simulink model "ClimaEgyCoorr"  (R2022b)
settings: Solver=FixedStepDiscrete  StartTime=0.0  StopTime=10.0  FixedStep=1  RelTol=1e-3
settings Model: Creator=blue.ge  LastModifiedBy=blue.ge
settings DataIOCC: SaveFormat=StructureWithTime
settings OptimizationCC: DefaultParameterBehavior=Inlined  EnhancedBackFolding=on  PassReuseOutputArgsAs=Individual arguments  ZeroExternalMemoryAtStartup=off  ZeroInternalMemoryAtStartup=off  OptimizeBlockOrder=speed  DifferentSizesBufferReuse=on  OptimizationCustomize=off  EfficientTunableParamExpr=on
settings DebuggingCC: ParameterTunabilityLossMsg=error
settings ModelReferenceCC: ModelReferenceNumInstancesAllowed=Single
settings SFSimCC: MATLABDynamicMemAlloc=off
settings RTWCC: Description=AUTOSAR  SystemTargetFile=autosar.tlc  GenCodeOnly=on  TemplateMakefile=ert_default_tmf
settings CodeAppCC: ForceParamTrailComments=on  IgnoreCustomStorageClasses=off  OperatorAnnotations=on  SimulinkDataObjDesc=on  SFDataObjDesc=on  InsertBlockDesc=on
settings STFCustomTargetCC: TargetLangStandard=C99 (ISO)  MultiwordLength=256  ParMdlRefBuildCompliant=on  CompOptLevelCompliant=on  IncludeMdlTerminateFcn=off  CombineOutputUpdateFcns=on  SuppressErrorStatus=on  ERTCustomFileBanners=on  SupportNonFinite=off  SupportComplex=off  SupportContinuousTime=off  SupportNonInlinedSFcns=off  RemoveResetFunc=on  MATLABClassNameForMDSCustomization=Simulink.SoftwareTarget.ERTCustomization  AutosarCompliant=on  UseToolchainInfoCompliant=on  LookupTableObjectStructAxisOrder=2,1,3,4,...  LUTObjectStructOrderEvenSpacing=Size,Table,Breakpoints  SystemTargetFile=autosar.tlc
settings ERTTargetCC: TargetLangStandard=C99 (ISO)  ConcurrentExecutionCompliant=off  IncludeMdlTerminateFcn=off  SuppressErrorStatus=on  SupportNonFinite=off  SupportComplex=off  ModelStepFunctionPrototypeControlCompliant=off  CPPClassGenCompliant=off  AutosarCompliant=on  LookupTableObjectStructAxisOrder=2,1,3,4,...  LUTObjectStructOrderEvenSpacing=Size,Table,Breakpoints  InlinedParameterPlacement=NonHierarchical  ERTCustomFileTemplate=example_file_process.tlc

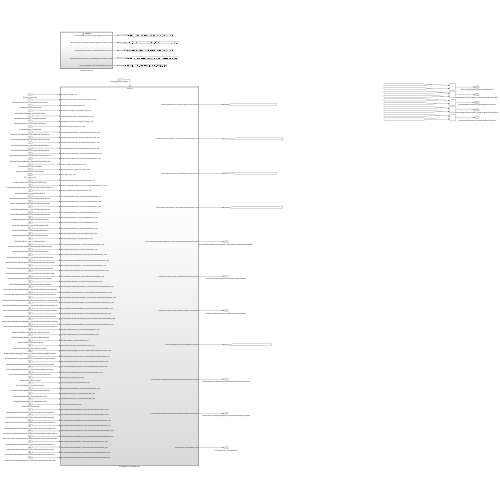
[thumbnail: Simulink's stored preview of the top-level diagram]
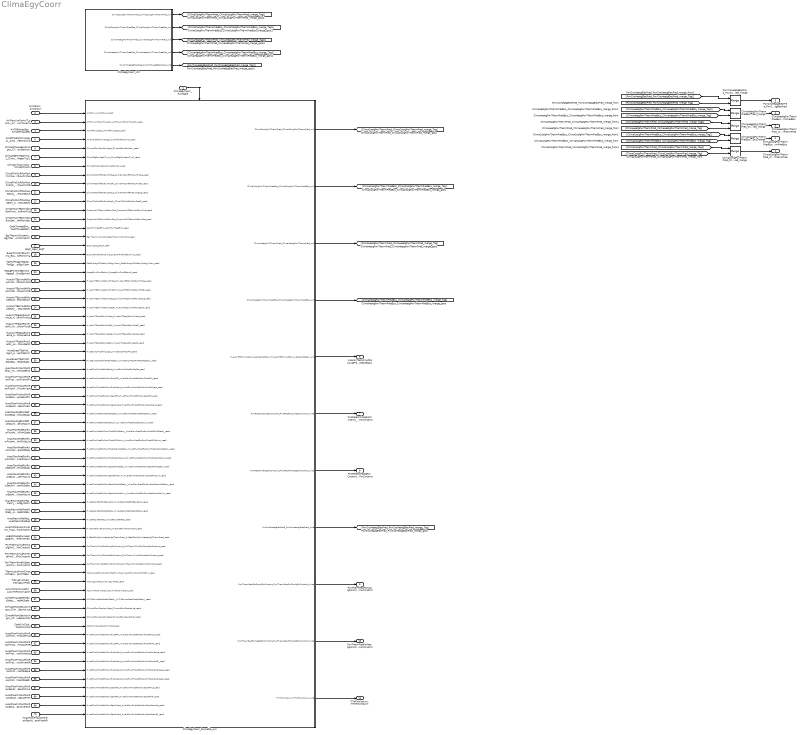
== system ClimaEgyCoorr ==
  ClimaEgyCoorr_Runnable : Inport  SampleTime=1  OutputFunctionCall=on
  AirCdnAvl_AirCdnAvl : Inport  Port=2  OutDataTypeStr=Boolean  PortDimensions=1
  AirFlowLimForAirTLoActv_AirFlowLimForAirTLoActv : Inport  Port=3  OutDataTypeStr=Boolean  PortDimensions=1
  AirTAftHvacDes_AirTAftHvacDes : Inport  Port=4  OutDataTypeStr=T30  PortDimensions=1
  AmbTFildForClimaNgcc_AmbTFildForClima : Inport  Port=5  OutDataTypeStr=T30  PortDimensions=1
  ClimaAtRowSecActvNgcc_ClimaAtRowSecActv : Inport  Port=6  OutDataTypeStr=Boolean  PortDimensions=1
  ClimaCfgForHeatrTyp1_ClimaCfgForHeatrTyp1 : Inport  Port=7  OutDataTypeStr=Enum: ClimaCfgForHeatrTyp1  PortDimensions=1
  ClimaCtrlActvNgcc_ClimaCtrlActv : Inport  Port=8  OutDataTypeStr=Boolean  PortDimensions=1
  ClimaTAtCmftForRowFirstLe_ClimaTAtCmftForRowFirstLe : Inport  Port=9  OutDataTypeStr=T30  PortDimensions=1
  ClimaTAtCmftForRowFirstRi_ClimaTAtCmftForRowFirstRi : Inport  Port=10  OutDataTypeStr=T30  PortDimensions=1
  ClimaTAtCmftForRowSecLe_ClimaTAtCmftForRowSecLe : Inport  Port=11  OutDataTypeStr=T30  PortDimensions=1
  ClimaTAtCmftForRowSecRi_ClimaTAtCmftForRowSecRi : Inport  Port=12  OutDataTypeStr=T30  PortDimensions=1
  CmptmtAirTEstimdAtRowFirst_CmptmtAirTEstimdAtRowFirst : Inport  Port=13  OutDataTypeStr=T30  PortDimensions=1
  CmptmtAirTEstimdAtRowSec_CmptmtAirTEstimdAtRowSec : Inport  Port=14  OutDataTypeStr=T30  PortDimensions=1
  CooltTInHeatElm_CooltTInHeatElm : Inport  Port=15  OutDataTypeStr=T30  PortDimensions=1
  EgyThermInCmptmt_EgyThermInCmptmt : Inport  Port=16  OutDataTypeStr=Egy33  PortDimensions=1
  EngT_Ngcc_EngT : Inport  Port=17  OutDataTypeStr=Bus: EngT  PortDimensions=1  BusOutputAsStruct=on
  EvaprTFrntFildForClima_EvaprTFrntFildForClima : Inport  Port=18  OutDataTypeStr=T30  PortDimensions=1
  FacForProgsToStabyForEgyCoorr_FacForProgsToStabyForEgyCoorr : Inport  Port=19  OutDataTypeStr=Fac30  PortDimensions=1
  HeatgPwrDmdEstimd_HeatgPwrDmdEstimd : Inport  Port=20  OutDataTypeStr=Pwr33  PortDimensions=1
  HvacAirTEstimdAtRowFirstLe_HvacAirTEstimdAtRowFirstLe : Inport  Port=21  OutDataTypeStr=T30  PortDimensions=1
  HvacAirTEstimdAtRowFirstRi_HvacAirTEstimdAtRowFirstRi : Inport  Port=22  OutDataTypeStr=T30  PortDimensions=1
  HvacAirTEstimdAtRowSecLe_HvacAirTEstimdAtRowSecLe : Inport  Port=23  OutDataTypeStr=T30  PortDimensions=1
  HvacAirTEstimdAtRowSecRi_HvacAirTEstimdAtRowSecRi : Inport  Port=24  OutDataTypeStr=T30  PortDimensions=1
  HvacAirTReqAtRowFirstLe_HvacAirTReqAtRowFirstLe : Inport  Port=25  OutDataTypeStr=T30  PortDimensions=1
  HvacAirTReqAtRowFirstRi_HvacAirTReqAtRowFirstRi : Inport  Port=26  OutDataTypeStr=T30  PortDimensions=1
  HvacAirTReqAtRowSecLe_HvacAirTReqAtRowSecLe : Inport  Port=27  OutDataTypeStr=T30  PortDimensions=1
  HvacAirTReqAtRowSecRi_HvacAirTReqAtRowSecRi : Inport  Port=28  OutDataTypeStr=T30  PortDimensions=1
  HvacEvaprTSpFrnt_Ngcc_HvacEvaprTSpFrnt : Inport  Port=29  OutDataTypeStr=HvacEvaprTSp  PortDimensions=1
  HvacEvaprTSpFrntPredAtStaby_HvacEvaprTSpFrntPredAtStaby : Inport  Port=30  OutDataTypeStr=HvacEvaprTSp  PortDimensions=1
  HvacFlowFrntActForDefrst_HvacFlowFrntActForDefrst : Inport  Port=31  OutDataTypeStr=AirFlow30  PortDimensions=1
  HvacFlowFrntActForRowFirstAtFlr_HvacFlowFrntActForRowFirstAtFlr : Inport  Port=32  OutDataTypeStr=AirFlow30  PortDimensions=1
  HvacFlowFrntActForRowFirstAtVent_HvacFlowFrntActForRowFirstAtVent : Inport  Port=33  OutDataTypeStr=AirFlow30  PortDimensions=1
  HvacFlowFrntActForRowSecAtFlr_HvacFlowFrntActForRowSecAtFlr : Inport  Port=34  OutDataTypeStr=AirFlow30  PortDimensions=1
  HvacFlowFrntActForRowSecAtVent_HvacFlowFrntActForRowSecAtVent : Inport  Port=35  OutDataTypeStr=AirFlow30  PortDimensions=1
  HvacFlowPredForDefrstAtStaby_HvacFlowPredForDefrstAtStaby : Inport  Port=36  OutDataTypeStr=AirFlow30  PortDimensions=1
  HvacFlowPredForDefrstNoLim_HvacFlowPredForDefrstNoLim : Inport  Port=37  OutDataTypeStr=AirFlow30  PortDimensions=1
  HvacFlowPredForRowFirstAtFlrAtStaby_HvacFlowPredForRowFirstAtFlrAtStaby : Inport  Port=38  OutDataTypeStr=AirFlow30  PortDimensions=1
  HvacFlowPredForRowFirstAtFlrNoLim_HvacFlowPredForRowFirstAtFlrNoLim : Inport  Port=39  OutDataTypeStr=AirFlow30  PortDimensions=1
  HvacFlowPredForRowFirstAtVentAtStaby_HvacFlowPredForRowFirstAtVentAtStaby : Inport  Port=40  OutDataTypeStr=AirFlow30  PortDimensions=1
  HvacFlowPredForRowFirstAtVentNoLim_HvacFlowPredForRowFirstAtVentNoLim : Inport  Port=41  OutDataTypeStr=AirFlow30  PortDimensions=1
  HvacFlowPredForRowSecAtFlrAtStaby_HvacFlowPredForRowSecAtFlrAtStaby : Inport  Port=42  OutDataTypeStr=AirFlow30  PortDimensions=1
  HvacFlowPredForRowSecAtFlrNoLim_HvacFlowPredForRowSecAtFlrNoLim : Inport  Port=43  OutDataTypeStr=AirFlow30  PortDimensions=1
  HvacFlowPredForRowSecAtVentAtStaby_HvacFlowPredForRowSecAtVentAtStaby : Inport  Port=44  OutDataTypeStr=AirFlow30  PortDimensions=1
  HvacFlowPredForRowSecAtVentNoLim_HvacFlowPredForRowSecAtVentNoLim : Inport  Port=45  OutDataTypeStr=AirFlow30  PortDimensions=1
  HvacRecircRatForEgyCalcn_HvacRecircRatForEgyCalcn : Inport  Port=46  OutDataTypeStr=Perc30  PortDimensions=1
  HvacRecircRatPredAtStaby_HvacRecircRatPredAtStaby : Inport  Port=47  OutDataTypeStr=Perc30  PortDimensions=1
  HvacRecircRatReq_HvacRecircRatReq : Inport  Port=48  OutDataTypeStr=Perc30  PortDimensions=1
  HvacTotFlowForCmptmt_HvacTotFlowForCmptmt : Inport  Port=49  OutDataTypeStr=AirFlow30  PortDimensions=1
  HvBattCoolgPwrHeatgMgrThermPred_HvBattCoolgPwrHeatgMgrThermPred : Inport  Port=50  OutDataTypeStr=Bus: HvBattCoolgPwrHeatgMgrThermPred  PortDimensions=1  BusOutputAsStruct=on
  PwrThermAllwdForCoolgForCmptmt_PwrThermAllwdForCoolgForCmptmt : Inport  Port=51  OutMin=0  OutMax=20460  OutDataTypeStr=Pwr3  PortDimensions=1
  PwrThermAllwdForHeatForCmptmt_PwrThermAllwdForHeatForCmptmt : Inport  Port=52  OutMin=0  OutMax=20460  OutDataTypeStr=Pwr3  PortDimensions=1
  PwrThermDmdAtStabyForCmptmt_PwrThermDmdAtStabyForCmptmt : Inport  Port=53  OutDataTypeStr=Pwr33  PortDimensions=1
  ThermLossFromCmptmtToEnv_ThermLossFromCmptmtToEnv : Inport  Port=54  OutDataTypeStr=Pwr33  PortDimensions=1
  TiDrvgCycPred_TiDrvgCycPred : Inport  Port=55  OutDataTypeStr=Ti43  PortDimensions=1
  ActvnTiForClimaCtrl_ActvnTiForClimaCtrl : Inport  Port=56  OutDataTypeStr=Ti33  PortDimensions=1
  AirTAftHvacDesPredAtStaby_AirTAftHvacDesPredAtStaby : Inport  Port=57  OutDataTypeStr=T30  PortDimensions=1
  ClimaAtRowSecActvNgcc_ClimaAtRowSecActvLe : Inport  Port=58  OutDataTypeStr=Boolean  PortDimensions=1
  ClimaAtRowSecActvNgcc_ClimaAtRowSecActvRi : Inport  Port=59  OutDataTypeStr=Boolean  PortDimensions=1
  CooltVlvClsd_CooltVlvClsd : Inport  Port=60  OutDataTypeStr=Boolean  PortDimensions=1
  HvacFlowFrntActForRowFirstAtFlr_HvacFlowFrntActForRowFirstAtFlrLe : Inport  Port=61  OutDataTypeStr=AirFlow30  PortDimensions=1
  HvacFlowFrntActForRowFirstAtFlr_HvacFlowFrntActForRowFirstAtFlrRi : Inport  Port=62  OutDataTypeStr=AirFlow30  PortDimensions=1
  HvacFlowFrntActForRowFirstAtVent_HvacFlowFrntActForRowFirstAtVentLe : Inport  Port=63  OutDataTypeStr=AirFlow30  PortDimensions=1
  HvacFlowFrntActForRowFirstAtVent_HvacFlowFrntActForRowFirstAtVentRi : Inport  Port=64  OutDataTypeStr=AirFlow30  PortDimensions=1
  HvacFlowFrntActForRowFirstAtVent_HvacFlowFrntActForRowFirstAtVentSideLe : Inport  Port=65  OutDataTypeStr=AirFlow30  PortDimensions=1
  HvacFlowFrntActForRowFirstAtVent_HvacFlowFrntActForRowFirstAtVentSideRi : Inport  Port=66  OutDataTypeStr=AirFlow30  PortDimensions=1
  HvacFlowFrntActForRowSecAtFlr_HvacFlowFrntActForRowSecAtFlrLe : Inport  Port=67  OutDataTypeStr=AirFlow30  PortDimensions=1
  HvacFlowFrntActForRowSecAtFlr_HvacFlowFrntActForRowSecAtFlrRi : Inport  Port=68  OutDataTypeStr=AirFlow30  PortDimensions=1
  HvacFlowFrntActForRowSecAtVent_HvacFlowFrntActForRowSecAtVentLe : Inport  Port=69  OutDataTypeStr=AirFlow30  PortDimensions=1
  HvacFlowFrntActForRowSecAtVent_HvacFlowFrntActForRowSecAtVentRi : Inport  Port=70  OutDataTypeStr=AirFlow30  PortDimensions=1
  ClimaCoolgPwrThermPredEco_ClimaCoolgPwrThermPredEco_merge : Merge
  ClimaCoolgPwrThermPred_ClimaCoolgPwrThermPred_merge : Merge
  ClimaEgyCoorr_Init : Subsystem
  ClimaEgyCoorr_Runnable_sys : Subsystem
  ClimaHeatgPwrThermPredEco_ClimaHeatgPwrThermPredEco_merge : Merge
  ClimaHeatgPwrThermPred_ClimaHeatgPwrThermPred_merge : Merge
  PwrCnsHeatgElecPred_PwrCnsHeatgElecPred_merge : Merge
  ClimaCoolgPwrThermPred_ClimaCoolgPwrThermPred : Outport  OutDataTypeStr=Bus: ClimaCoolgPwrThermPred  PortDimensions=1  BusOutputAsStruct=on
  ClimaCoolgPwrThermPredEco_ClimaCoolgPwrThermPredEco : Outport  Port=2  OutDataTypeStr=Bus: ClimaCoolgPwrThermPredEcoRec  PortDimensions=1  BusOutputAsStruct=on
  ClimaHeatgPwrThermPred_ClimaHeatgPwrThermPred : Outport  Port=3  OutDataTypeStr=Bus: ClimaHeatgPwrThermPred  PortDimensions=1  BusOutputAsStruct=on
  ClimaHeatgPwrThermPredEco_ClimaHeatgPwrThermPredEco : Outport  Port=4  OutDataTypeStr=Bus: ClimaHeatgPwrThermPredEcoRec  PortDimensions=1  BusOutputAsStruct=on
  PwrCnsHeatgElecPred_PwrCnsHeatgElecPred : Outport  Port=5  OutDataTypeStr=Bus: PwrCnsHeatgElecPred  PortDimensions=1  BusOutputAsStruct=on
  HvacAirTEstimnAtRowAvgPredAtStaby_HvacAirTEstimnAtRowAvgPredAtStaby : Outport  Port=6  OutDataTypeStr=T30  PortDimensions=1
  PrioReqForCoolgForCmptmt_PrioReqForCoolgForCmptmt : Outport  Port=7  OutDataTypeStr=Enum: LvlOfPrioTyp  PortDimensions=1
  PrioReqForHeatgForCmptmt_PrioReqForHeatgForCmptmt : Outport  Port=8  OutDataTypeStr=Enum: LvlOfPrioTyp  PortDimensions=1
  PwrThermReqForCoolgForCmptmt_PwrThermReqForCoolgForCmptmt : Outport  Port=9  OutMin=0  OutMax=20460  OutDataTypeStr=Pwr3  PortDimensions=1
  PwrThermReqForHeatgForCmptmt_PwrThermReqForHeatgForCmptmt : Outport  Port=10  OutMin=0  OutMax=20460  OutDataTypeStr=Pwr3  PortDimensions=1
  TiToFlowNoLim_TiToFlowNoLim : Outport  Port=11  OutDataTypeStr=Ti33  PortDimensions=1
  wires:
  AirCdnAvl_AirCdnAvl/1 -> ClimaEgyCoorr_Runnable_sys/1(AirCdnAvl_AirCdnAvl_read)
  AirFlowLimForAirTLoActv_AirFlowLimForAirTLoActv/1 -> ClimaEgyCoorr_Runnable_sys/2(AirFlowLimForAirTLoActv_AirFlowLimForAirTLoActv_read)
  AirTAftHvacDes_AirTAftHvacDes/1 -> ClimaEgyCoorr_Runnable_sys/3(AirTAftHvacDes_AirTAftHvacDes_read)
  AmbTFildForClimaNgcc_AmbTFildForClima/1 -> ClimaEgyCoorr_Runnable_sys/4(AmbTFildForClimaNgcc_AmbTFildForClima_read)
  ClimaAtRowSecActvNgcc_ClimaAtRowSecActv/1 -> ClimaEgyCoorr_Runnable_sys/5(ClimaAtRowSecActvNgcc_ClimaAtRowSecActv_read)
  ClimaCfgForHeatrTyp1_ClimaCfgForHeatrTyp1/1 -> ClimaEgyCoorr_Runnable_sys/6(ClimaCfgForHeatrTyp1_ClimaCfgForHeatrTyp1_read)
  ClimaCtrlActvNgcc_ClimaCtrlActv/1 -> ClimaEgyCoorr_Runnable_sys/7(ClimaCtrlActvNgcc_ClimaCtrlActv_read)
  ClimaTAtCmftForRowFirstLe_ClimaTAtCmftForRowFirstLe/1 -> ClimaEgyCoorr_Runnable_sys/8(ClimaTAtCmftForRowFirstLe_ClimaTAtCmftForRowFirstLe_read)
  ClimaTAtCmftForRowFirstRi_ClimaTAtCmftForRowFirstRi/1 -> ClimaEgyCoorr_Runnable_sys/9(ClimaTAtCmftForRowFirstRi_ClimaTAtCmftForRowFirstRi_read)
  ClimaTAtCmftForRowSecLe_ClimaTAtCmftForRowSecLe/1 -> ClimaEgyCoorr_Runnable_sys/10(ClimaTAtCmftForRowSecLe_ClimaTAtCmftForRowSecLe_read)
  ClimaTAtCmftForRowSecRi_ClimaTAtCmftForRowSecRi/1 -> ClimaEgyCoorr_Runnable_sys/11(ClimaTAtCmftForRowSecRi_ClimaTAtCmftForRowSecRi_read)
  CmptmtAirTEstimdAtRowFirst_CmptmtAirTEstimdAtRowFirst/1 -> ClimaEgyCoorr_Runnable_sys/12(CmptmtAirTEstimdAtRowFirst_CmptmtAirTEstimdAtRowFirst_read)
  CmptmtAirTEstimdAtRowSec_CmptmtAirTEstimdAtRowSec/1 -> ClimaEgyCoorr_Runnable_sys/13(CmptmtAirTEstimdAtRowSec_CmptmtAirTEstimdAtRowSec_read)
  CooltTInHeatElm_CooltTInHeatElm/1 -> ClimaEgyCoorr_Runnable_sys/14(CooltTInHeatElm_CooltTInHeatElm_read)
  EgyThermInCmptmt_EgyThermInCmptmt/1 -> ClimaEgyCoorr_Runnable_sys/15(EgyThermInCmptmt_EgyThermInCmptmt_read)
  EngT_Ngcc_EngT/1 -> ClimaEgyCoorr_Runnable_sys/16(EngT_Ngcc_EngT_read)
  EvaprTFrntFildForClima_EvaprTFrntFildForClima/1 -> ClimaEgyCoorr_Runnable_sys/17(EvaprTFrntFildForClima_EvaprTFrntFildForClima_read)
  FacForProgsToStabyForEgyCoorr_FacForProgsToStabyForEgyCoorr/1 -> ClimaEgyCoorr_Runnable_sys/18(FacForProgsToStabyForEgyCoorr_FacForProgsToStabyForEgyCoorr_read)
  HeatgPwrDmdEstimd_HeatgPwrDmdEstimd/1 -> ClimaEgyCoorr_Runnable_sys/19(HeatgPwrDmdEstimd_HeatgPwrDmdEstimd_read)
  HvacAirTEstimdAtRowFirstLe_HvacAirTEstimdAtRowFirstLe/1 -> ClimaEgyCoorr_Runnable_sys/20(HvacAirTEstimdAtRowFirstLe_HvacAirTEstimdAtRowFirstLe_read)
  HvacAirTEstimdAtRowFirstRi_HvacAirTEstimdAtRowFirstRi/1 -> ClimaEgyCoorr_Runnable_sys/21(HvacAirTEstimdAtRowFirstRi_HvacAirTEstimdAtRowFirstRi_read)
  HvacAirTEstimdAtRowSecLe_HvacAirTEstimdAtRowSecLe/1 -> ClimaEgyCoorr_Runnable_sys/22(HvacAirTEstimdAtRowSecLe_HvacAirTEstimdAtRowSecLe_read)
  HvacAirTEstimdAtRowSecRi_HvacAirTEstimdAtRowSecRi/1 -> ClimaEgyCoorr_Runnable_sys/23(HvacAirTEstimdAtRowSecRi_HvacAirTEstimdAtRowSecRi_read)
  HvacAirTReqAtRowFirstLe_HvacAirTReqAtRowFirstLe/1 -> ClimaEgyCoorr_Runnable_sys/24(HvacAirTReqAtRowFirstLe_HvacAirTReqAtRowFirstLe_read)
  HvacAirTReqAtRowFirstRi_HvacAirTReqAtRowFirstRi/1 -> ClimaEgyCoorr_Runnable_sys/25(HvacAirTReqAtRowFirstRi_HvacAirTReqAtRowFirstRi_read)
  HvacAirTReqAtRowSecLe_HvacAirTReqAtRowSecLe/1 -> ClimaEgyCoorr_Runnable_sys/26(HvacAirTReqAtRowSecLe_HvacAirTReqAtRowSecLe_read)
  HvacAirTReqAtRowSecRi_HvacAirTReqAtRowSecRi/1 -> ClimaEgyCoorr_Runnable_sys/27(HvacAirTReqAtRowSecRi_HvacAirTReqAtRowSecRi_read)
  HvacEvaprTSpFrnt_Ngcc_HvacEvaprTSpFrnt/1 -> ClimaEgyCoorr_Runnable_sys/28(HvacEvaprTSpFrnt_Ngcc_HvacEvaprTSpFrnt_read)
  HvacEvaprTSpFrntPredAtStaby_HvacEvaprTSpFrntPredAtStaby/1 -> ClimaEgyCoorr_Runnable_sys/29(HvacEvaprTSpFrntPredAtStaby_HvacEvaprTSpFrntPredAtStaby_read)
  HvacFlowFrntActForDefrst_HvacFlowFrntActForDefrst/1 -> ClimaEgyCoorr_Runnable_sys/30(HvacFlowFrntActForDefrst_HvacFlowFrntActForDefrst_read)
  HvacFlowFrntActForRowFirstAtFlr_HvacFlowFrntActForRowFirstAtFlr/1 -> ClimaEgyCoorr_Runnable_sys/31(HvacFlowFrntActForRowFirstAtFlr_HvacFlowFrntActForRowFirstAtFlr_read)
  HvacFlowFrntActForRowFirstAtVent_HvacFlowFrntActForRowFirstAtVent/1 -> ClimaEgyCoorr_Runnable_sys/32(HvacFlowFrntActForRowFirstAtVent_HvacFlowFrntActForRowFirstAtVent_read)
  HvacFlowFrntActForRowSecAtFlr_HvacFlowFrntActForRowSecAtFlr/1 -> ClimaEgyCoorr_Runnable_sys/33(HvacFlowFrntActForRowSecAtFlr_HvacFlowFrntActForRowSecAtFlr_read)
  HvacFlowFrntActForRowSecAtVent_HvacFlowFrntActForRowSecAtVent/1 -> ClimaEgyCoorr_Runnable_sys/34(HvacFlowFrntActForRowSecAtVent_HvacFlowFrntActForRowSecAtVent_read)
  HvacFlowPredForDefrstAtStaby_HvacFlowPredForDefrstAtStaby/1 -> ClimaEgyCoorr_Runnable_sys/35(HvacFlowPredForDefrstAtStaby_HvacFlowPredForDefrstAtStaby_read)
  HvacFlowPredForDefrstNoLim_HvacFlowPredForDefrstNoLim/1 -> ClimaEgyCoorr_Runnable_sys/36(HvacFlowPredForDefrstNoLim_HvacFlowPredForDefrstNoLim_read)
  HvacFlowPredForRowFirstAtFlrAtStaby_HvacFlowPredForRowFirstAtFlrAtStaby/1 -> ClimaEgyCoorr_Runnable_sys/37(HvacFlowPredForRowFirstAtFlrAtStaby_HvacFlowPredForRowFirstAtFlrAtStaby_read)
  HvacFlowPredForRowFirstAtFlrNoLim_HvacFlowPredForRowFirstAtFlrNoLim/1 -> ClimaEgyCoorr_Runnable_sys/38(HvacFlowPredForRowFirstAtFlrNoLim_HvacFlowPredForRowFirstAtFlrNoLim_read)
  HvacFlowPredForRowFirstAtVentAtStaby_HvacFlowPredForRowFirstAtVentAtStaby/1 -> ClimaEgyCoorr_Runnable_sys/39(HvacFlowPredForRowFirstAtVentAtStaby_HvacFlowPredForRowFirstAtVentAtStaby_read)
  HvacFlowPredForRowFirstAtVentNoLim_HvacFlowPredForRowFirstAtVentNoLim/1 -> ClimaEgyCoorr_Runnable_sys/40(HvacFlowPredForRowFirstAtVentNoLim_HvacFlowPredForRowFirstAtVentNoLim_read)
  HvacFlowPredForRowSecAtFlrAtStaby_HvacFlowPredForRowSecAtFlrAtStaby/1 -> ClimaEgyCoorr_Runnable_sys/41(HvacFlowPredForRowSecAtFlrAtStaby_HvacFlowPredForRowSecAtFlrAtStaby_read)
  HvacFlowPredForRowSecAtFlrNoLim_HvacFlowPredForRowSecAtFlrNoLim/1 -> ClimaEgyCoorr_Runnable_sys/42(HvacFlowPredForRowSecAtFlrNoLim_HvacFlowPredForRowSecAtFlrNoLim_read)
  HvacFlowPredForRowSecAtVentAtStaby_HvacFlowPredForRowSecAtVentAtStaby/1 -> ClimaEgyCoorr_Runnable_sys/43(HvacFlowPredForRowSecAtVentAtStaby_HvacFlowPredForRowSecAtVentAtStaby_read)
  HvacFlowPredForRowSecAtVentNoLim_HvacFlowPredForRowSecAtVentNoLim/1 -> ClimaEgyCoorr_Runnable_sys/44(HvacFlowPredForRowSecAtVentNoLim_HvacFlowPredForRowSecAtVentNoLim_read)
  HvacRecircRatForEgyCalcn_HvacRecircRatForEgyCalcn/1 -> ClimaEgyCoorr_Runnable_sys/45(HvacRecircRatForEgyCalcn_HvacRecircRatForEgyCalcn_read)
  HvacRecircRatPredAtStaby_HvacRecircRatPredAtStaby/1 -> ClimaEgyCoorr_Runnable_sys/46(HvacRecircRatPredAtStaby_HvacRecircRatPredAtStaby_read)
  HvacRecircRatReq_HvacRecircRatReq/1 -> ClimaEgyCoorr_Runnable_sys/47(HvacRecircRatReq_HvacRecircRatReq_read)
  HvacTotFlowForCmptmt_HvacTotFlowForCmptmt/1 -> ClimaEgyCoorr_Runnable_sys/48(HvacTotFlowForCmptmt_HvacTotFlowForCmptmt_read)
  HvBattCoolgPwrHeatgMgrThermPred_HvBattCoolgPwrHeatgMgrThermPred/1 -> ClimaEgyCoorr_Runnable_sys/49(HvBattCoolgPwrHeatgMgrThermPred_HvBattCoolgPwrHeatgMgrThermPred_read)
  PwrThermAllwdForCoolgForCmptmt_PwrThermAllwdForCoolgForCmptmt/1 -> ClimaEgyCoorr_Runnable_sys/50(PwrThermAllwdForCoolgForCmptmt_PwrThermAllwdForCoolgForCmptmt_read)
  PwrThermAllwdForHeatForCmptmt_PwrThermAllwdForHeatForCmptmt/1 -> ClimaEgyCoorr_Runnable_sys/51(PwrThermAllwdForHeatForCmptmt_PwrThermAllwdForHeatForCmptmt_read)
  PwrThermDmdAtStabyForCmptmt_PwrThermDmdAtStabyForCmptmt/1 -> ClimaEgyCoorr_Runnable_sys/52(PwrThermDmdAtStabyForCmptmt_PwrThermDmdAtStabyForCmptmt_read)
  ThermLossFromCmptmtToEnv_ThermLossFromCmptmtToEnv/1 -> ClimaEgyCoorr_Runnable_sys/53(ThermLossFromCmptmtToEnv_ThermLossFromCmptmtToEnv_read)
  TiDrvgCycPred_TiDrvgCycPred/1 -> ClimaEgyCoorr_Runnable_sys/54(TiDrvgCycPred_TiDrvgCycPred_read)
  ActvnTiForClimaCtrl_ActvnTiForClimaCtrl/1 -> ClimaEgyCoorr_Runnable_sys/55(ActvnTiForClimaCtrl_ActvnTiForClimaCtrl_read)
  AirTAftHvacDesPredAtStaby_AirTAftHvacDesPredAtStaby/1 -> ClimaEgyCoorr_Runnable_sys/56(AirTAftHvacDesPredAtStaby_AirTAftHvacDesPredAtStaby_read)
  ClimaAtRowSecActvNgcc_ClimaAtRowSecActvLe/1 -> ClimaEgyCoorr_Runnable_sys/57(ClimaAtRowSecActvNgcc_ClimaAtRowSecActvLe_read)
  ClimaAtRowSecActvNgcc_ClimaAtRowSecActvRi/1 -> ClimaEgyCoorr_Runnable_sys/58(ClimaAtRowSecActvNgcc_ClimaAtRowSecActvRi_read)
  CooltVlvClsd_CooltVlvClsd/1 -> ClimaEgyCoorr_Runnable_sys/59(CooltVlvClsd_CooltVlvClsd_read)
  HvacFlowFrntActForRowFirstAtFlr_HvacFlowFrntActForRowFirstAtFlrLe/1 -> ClimaEgyCoorr_Runnable_sys/60(HvacFlowFrntActForRowFirstAtFlr_HvacFlowFrntActForRowFirstAtFlrLe_read)
  HvacFlowFrntActForRowFirstAtFlr_HvacFlowFrntActForRowFirstAtFlrRi/1 -> ClimaEgyCoorr_Runnable_sys/61(HvacFlowFrntActForRowFirstAtFlr_HvacFlowFrntActForRowFirstAtFlrRi_read)
  HvacFlowFrntActForRowFirstAtVent_HvacFlowFrntActForRowFirstAtVentLe/1 -> ClimaEgyCoorr_Runnable_sys/62(HvacFlowFrntActForRowFirstAtVent_HvacFlowFrntActForRowFirstAtVentLe_read)
  HvacFlowFrntActForRowFirstAtVent_HvacFlowFrntActForRowFirstAtVentRi/1 -> ClimaEgyCoorr_Runnable_sys/63(HvacFlowFrntActForRowFirstAtVent_HvacFlowFrntActForRowFirstAtVentRi_read)
  HvacFlowFrntActForRowFirstAtVent_HvacFlowFrntActForRowFirstAtVentSideLe/1 -> ClimaEgyCoorr_Runnable_sys/64(HvacFlowFrntActForRowFirstAtVent_HvacFlowFrntActForRowFirstAtVentSideLe_read)
  HvacFlowFrntActForRowFirstAtVent_HvacFlowFrntActForRowFirstAtVentSideRi/1 -> ClimaEgyCoorr_Runnable_sys/65(HvacFlowFrntActForRowFirstAtVent_HvacFlowFrntActForRowFirstAtVentSideRi_read)
  HvacFlowFrntActForRowSecAtFlr_HvacFlowFrntActForRowSecAtFlrLe/1 -> ClimaEgyCoorr_Runnable_sys/66(HvacFlowFrntActForRowSecAtFlr_HvacFlowFrntActForRowSecAtFlrLe_read)
  HvacFlowFrntActForRowSecAtFlr_HvacFlowFrntActForRowSecAtFlrRi/1 -> ClimaEgyCoorr_Runnable_sys/67(HvacFlowFrntActForRowSecAtFlr_HvacFlowFrntActForRowSecAtFlrRi_read)
  HvacFlowFrntActForRowSecAtVent_HvacFlowFrntActForRowSecAtVentLe/1 -> ClimaEgyCoorr_Runnable_sys/68(HvacFlowFrntActForRowSecAtVent_HvacFlowFrntActForRowSecAtVentLe_read)
  HvacFlowFrntActForRowSecAtVent_HvacFlowFrntActForRowSecAtVentRi/1 -> ClimaEgyCoorr_Runnable_sys/69(HvacFlowFrntActForRowSecAtVent_HvacFlowFrntActForRowSecAtVentRi_read)
  ClimaCoolgPwrThermPred_ClimaCoolgPwrThermPred_merge/1 -> ClimaCoolgPwrThermPred_ClimaCoolgPwrThermPred/1
  ClimaCoolgPwrThermPredEco_ClimaCoolgPwrThermPredEco_merge/1 -> ClimaCoolgPwrThermPredEco_ClimaCoolgPwrThermPredEco/1
  ClimaHeatgPwrThermPred_ClimaHeatgPwrThermPred_merge/1 -> ClimaHeatgPwrThermPred_ClimaHeatgPwrThermPred/1
  ClimaHeatgPwrThermPredEco_ClimaHeatgPwrThermPredEco_merge/1 -> ClimaHeatgPwrThermPredEco_ClimaHeatgPwrThermPredEco/1
  ClimaEgyCoorr_Runnable_sys/5(HvacAirTEstimnAtRowAvgPredAtStaby_HvacAirTEstimnAtRowAvgPredAtStaby_write) -> HvacAirTEstimnAtRowAvgPredAtStaby_HvacAirTEstimnAtRowAvgPredAtStaby/1
  ClimaEgyCoorr_Runnable_sys/6(PrioReqForCoolgForCmptmt_PrioReqForCoolgForCmptmt_write) -> PrioReqForCoolgForCmptmt_PrioReqForCoolgForCmptmt/1
  ClimaEgyCoorr_Runnable_sys/7(PrioReqForHeatgForCmptmt_PrioReqForHeatgForCmptmt_write) -> PrioReqForHeatgForCmptmt_PrioReqForHeatgForCmptmt/1
  PwrCnsHeatgElecPred_PwrCnsHeatgElecPred_merge/1 -> PwrCnsHeatgElecPred_PwrCnsHeatgElecPred/1
  ClimaEgyCoorr_Runnable_sys/9(PwrThermReqForCoolgForCmptmt_PwrThermReqForCoolgForCmptmt_write) -> PwrThermReqForCoolgForCmptmt_PwrThermReqForCoolgForCmptmt/1
  ClimaEgyCoorr_Runnable_sys/10(PwrThermReqForHeatgForCmptmt_PwrThermReqForHeatgForCmptmt_write) -> PwrThermReqForHeatgForCmptmt_PwrThermReqForHeatgForCmptmt/1
  ClimaEgyCoorr_Runnable_sys/11(TiToFlowNoLim_TiToFlowNoLim_write) -> TiToFlowNoLim_TiToFlowNoLim/1
  ClimaEgyCoorr_Runnable_sys/8(PwrCnsHeatgElecPred_PwrCnsHeatgElecPred_write) -> [PwrCnsHeatgElecPred_PwrCnsHeatgElecPred_merge_Tag]
  [PwrCnsHeatgElecPred_PwrCnsHeatgElecPred_merge_Tag] -> PwrCnsHeatgElecPred_PwrCnsHeatgElecPred_merge/2
  ClimaEgyCoorr_Init/5(PwrCnsHeatgElecPred_PwrCnsHeatgElecPred_write) -> [PwrCnsHeatgElecPred_PwrCnsHeatgElecPred_merge_Tag1]
  [PwrCnsHeatgElecPred_PwrCnsHeatgElecPred_merge_Tag1] -> PwrCnsHeatgElecPred_PwrCnsHeatgElecPred_merge/1
  ClimaEgyCoorr_Runnable_sys/4(ClimaHeatgPwrThermPredEco_ClimaHeatgPwrThermPredEco_write) -> [ClimaHeatgPwrThermPredEco_ClimaHeatgPwrThermPredEco_merge_Tag]
  [ClimaHeatgPwrThermPredEco_ClimaHeatgPwrThermPredEco_merge_Tag] -> ClimaHeatgPwrThermPredEco_ClimaHeatgPwrThermPredEco_merge/2
  ClimaEgyCoorr_Init/4(ClimaHeatgPwrThermPredEco_ClimaHeatgPwrThermPredEco_write) -> [ClimaHeatgPwrThermPredEco_ClimaHeatgPwrThermPredEco_merge_Tag1]
  [ClimaHeatgPwrThermPredEco_ClimaHeatgPwrThermPredEco_merge_Tag1] -> ClimaHeatgPwrThermPredEco_ClimaHeatgPwrThermPredEco_merge/1
  ClimaEgyCoorr_Runnable_sys/3(ClimaHeatgPwrThermPred_ClimaHeatgPwrThermPred_write) -> [ClimaHeatgPwrThermPred_ClimaHeatgPwrThermPred_merge_Tag]
  [ClimaHeatgPwrThermPred_ClimaHeatgPwrThermPred_merge_Tag] -> ClimaHeatgPwrThermPred_ClimaHeatgPwrThermPred_merge/2
  ClimaEgyCoorr_Init/3(ClimaHeatgPwrThermPred_ClimaHeatgPwrThermPred_write) -> [ClimaHeatgPwrThermPred_ClimaHeatgPwrThermPred_merge_Tag1]
  [ClimaHeatgPwrThermPred_ClimaHeatgPwrThermPred_merge_Tag1] -> ClimaHeatgPwrThermPred_ClimaHeatgPwrThermPred_merge/1
  ClimaEgyCoorr_Runnable_sys/2(ClimaCoolgPwrThermPredEco_ClimaCoolgPwrThermPredEco_write) -> [ClimaCoolgPwrThermPredEco_ClimaCoolgPwrThermPredEco_merge_Tag]
  [ClimaCoolgPwrThermPredEco_ClimaCoolgPwrThermPredEco_merge_Tag] -> ClimaCoolgPwrThermPredEco_ClimaCoolgPwrThermPredEco_merge/2
  ClimaEgyCoorr_Init/2(ClimaCoolgPwrThermPredEco_ClimaCoolgPwrThermPredEco_write) -> [ClimaCoolgPwrThermPredEco_ClimaCoolgPwrThermPredEco_merge_Tag1]
  [ClimaCoolgPwrThermPredEco_ClimaCoolgPwrThermPredEco_merge_Tag1] -> ClimaCoolgPwrThermPredEco_ClimaCoolgPwrThermPredEco_merge/1
  ClimaEgyCoorr_Runnable_sys/1(ClimaCoolgPwrThermPred_ClimaCoolgPwrThermPred_write) -> [ClimaCoolgPwrThermPred_ClimaCoolgPwrThermPred_merge_Tag]
  [ClimaCoolgPwrThermPred_ClimaCoolgPwrThermPred_merge_Tag] -> ClimaCoolgPwrThermPred_ClimaCoolgPwrThermPred_merge/2
  ClimaEgyCoorr_Init/1(ClimaCoolgPwrThermPred_ClimaCoolgPwrThermPred_write) -> [ClimaCoolgPwrThermPred_ClimaCoolgPwrThermPred_merge_Tag1]
  [ClimaCoolgPwrThermPred_ClimaCoolgPwrThermPred_merge_Tag1] -> ClimaCoolgPwrThermPred_ClimaCoolgPwrThermPred_merge/1
  ClimaEgyCoorr_Runnable/1 -> ClimaEgyCoorr_Runnable_sys/Trigger

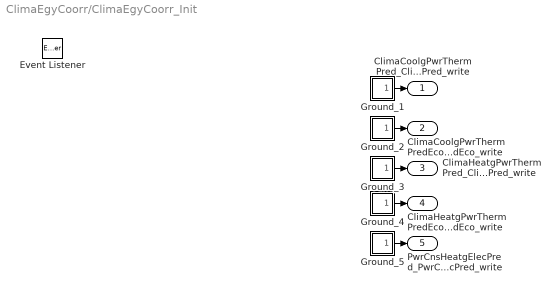
== system ClimaEgyCoorr/ClimaEgyCoorr_Init ==
  Event Listener : EventListener  EventName=reset
  Ground_1 : Subsystem
  Ground_2 : Subsystem
  Ground_3 : Subsystem
  Ground_4 : Subsystem
  Ground_5 : Subsystem
  ClimaCoolgPwrThermPred_ClimaCoolgPwrThermPred_write : Outport
  ClimaCoolgPwrThermPredEco_ClimaCoolgPwrThermPredEco_write : Outport  Port=2
  ClimaHeatgPwrThermPred_ClimaHeatgPwrThermPred_write : Outport  Port=3
  ClimaHeatgPwrThermPredEco_ClimaHeatgPwrThermPredEco_write : Outport  Port=4
  PwrCnsHeatgElecPred_PwrCnsHeatgElecPred_write : Outport  Port=5
  wires:
  Ground_1/1(Out1) -> ClimaCoolgPwrThermPred_ClimaCoolgPwrThermPred_write/1
  Ground_2/1(Out1) -> ClimaCoolgPwrThermPredEco_ClimaCoolgPwrThermPredEco_write/1
  Ground_3/1(Out1) -> ClimaHeatgPwrThermPred_ClimaHeatgPwrThermPred_write/1
  Ground_4/1(Out1) -> ClimaHeatgPwrThermPredEco_ClimaHeatgPwrThermPredEco_write/1
  Ground_5/1(Out1) -> PwrCnsHeatgElecPred_PwrCnsHeatgElecPred_write/1

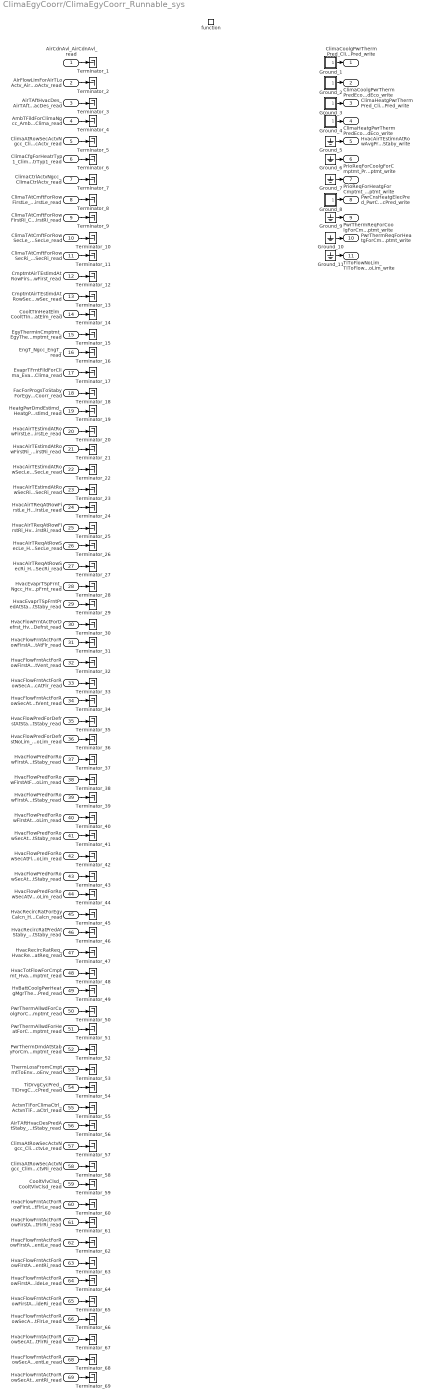
== system ClimaEgyCoorr/ClimaEgyCoorr_Runnable_sys ==
  AirCdnAvl_AirCdnAvl_read : Inport
  AirFlowLimForAirTLoActv_AirFlowLimForAirTLoActv_read : Inport  Port=2
  AirTAftHvacDes_AirTAftHvacDes_read : Inport  Port=3
  AmbTFildForClimaNgcc_AmbTFildForClima_read : Inport  Port=4
  ClimaAtRowSecActvNgcc_ClimaAtRowSecActv_read : Inport  Port=5
  ClimaCfgForHeatrTyp1_ClimaCfgForHeatrTyp1_read : Inport  Port=6
  ClimaCtrlActvNgcc_ClimaCtrlActv_read : Inport  Port=7
  ClimaTAtCmftForRowFirstLe_ClimaTAtCmftForRowFirstLe_read : Inport  Port=8
  ClimaTAtCmftForRowFirstRi_ClimaTAtCmftForRowFirstRi_read : Inport  Port=9
  ClimaTAtCmftForRowSecLe_ClimaTAtCmftForRowSecLe_read : Inport  Port=10
  ClimaTAtCmftForRowSecRi_ClimaTAtCmftForRowSecRi_read : Inport  Port=11
  CmptmtAirTEstimdAtRowFirst_CmptmtAirTEstimdAtRowFirst_read : Inport  Port=12
  CmptmtAirTEstimdAtRowSec_CmptmtAirTEstimdAtRowSec_read : Inport  Port=13
  CooltTInHeatElm_CooltTInHeatElm_read : Inport  Port=14
  EgyThermInCmptmt_EgyThermInCmptmt_read : Inport  Port=15
  EngT_Ngcc_EngT_read : Inport  Port=16
  EvaprTFrntFildForClima_EvaprTFrntFildForClima_read : Inport  Port=17
  FacForProgsToStabyForEgyCoorr_FacForProgsToStabyForEgyCoorr_read : Inport  Port=18
  HeatgPwrDmdEstimd_HeatgPwrDmdEstimd_read : Inport  Port=19
  HvacAirTEstimdAtRowFirstLe_HvacAirTEstimdAtRowFirstLe_read : Inport  Port=20
  HvacAirTEstimdAtRowFirstRi_HvacAirTEstimdAtRowFirstRi_read : Inport  Port=21
  HvacAirTEstimdAtRowSecLe_HvacAirTEstimdAtRowSecLe_read : Inport  Port=22
  HvacAirTEstimdAtRowSecRi_HvacAirTEstimdAtRowSecRi_read : Inport  Port=23
  HvacAirTReqAtRowFirstLe_HvacAirTReqAtRowFirstLe_read : Inport  Port=24
  HvacAirTReqAtRowFirstRi_HvacAirTReqAtRowFirstRi_read : Inport  Port=25
  HvacAirTReqAtRowSecLe_HvacAirTReqAtRowSecLe_read : Inport  Port=26
  HvacAirTReqAtRowSecRi_HvacAirTReqAtRowSecRi_read : Inport  Port=27
  HvacEvaprTSpFrnt_Ngcc_HvacEvaprTSpFrnt_read : Inport  Port=28
  HvacEvaprTSpFrntPredAtStaby_HvacEvaprTSpFrntPredAtStaby_read : Inport  Port=29
  HvacFlowFrntActForDefrst_HvacFlowFrntActForDefrst_read : Inport  Port=30
  HvacFlowFrntActForRowFirstAtFlr_HvacFlowFrntActForRowFirstAtFlr_read : Inport  Port=31
  HvacFlowFrntActForRowFirstAtVent_HvacFlowFrntActForRowFirstAtVent_read : Inport  Port=32
  HvacFlowFrntActForRowSecAtFlr_HvacFlowFrntActForRowSecAtFlr_read : Inport  Port=33
  HvacFlowFrntActForRowSecAtVent_HvacFlowFrntActForRowSecAtVent_read : Inport  Port=34
  HvacFlowPredForDefrstAtStaby_HvacFlowPredForDefrstAtStaby_read : Inport  Port=35
  HvacFlowPredForDefrstNoLim_HvacFlowPredForDefrstNoLim_read : Inport  Port=36
  HvacFlowPredForRowFirstAtFlrAtStaby_HvacFlowPredForRowFirstAtFlrAtStaby_read : Inport  Port=37
  HvacFlowPredForRowFirstAtFlrNoLim_HvacFlowPredForRowFirstAtFlrNoLim_read : Inport  Port=38
  HvacFlowPredForRowFirstAtVentAtStaby_HvacFlowPredForRowFirstAtVentAtStaby_read : Inport  Port=39
  HvacFlowPredForRowFirstAtVentNoLim_HvacFlowPredForRowFirstAtVentNoLim_read : Inport  Port=40
  HvacFlowPredForRowSecAtFlrAtStaby_HvacFlowPredForRowSecAtFlrAtStaby_read : Inport  Port=41
  HvacFlowPredForRowSecAtFlrNoLim_HvacFlowPredForRowSecAtFlrNoLim_read : Inport  Port=42
  HvacFlowPredForRowSecAtVentAtStaby_HvacFlowPredForRowSecAtVentAtStaby_read : Inport  Port=43
  HvacFlowPredForRowSecAtVentNoLim_HvacFlowPredForRowSecAtVentNoLim_read : Inport  Port=44
  HvacRecircRatForEgyCalcn_HvacRecircRatForEgyCalcn_read : Inport  Port=45
  HvacRecircRatPredAtStaby_HvacRecircRatPredAtStaby_read : Inport  Port=46
  HvacRecircRatReq_HvacRecircRatReq_read : Inport  Port=47
  HvacTotFlowForCmptmt_HvacTotFlowForCmptmt_read : Inport  Port=48
  HvBattCoolgPwrHeatgMgrThermPred_HvBattCoolgPwrHeatgMgrThermPred_read : Inport  Port=49
  PwrThermAllwdForCoolgForCmptmt_PwrThermAllwdForCoolgForCmptmt_read : Inport  Port=50
  PwrThermAllwdForHeatForCmptmt_PwrThermAllwdForHeatForCmptmt_read : Inport  Port=51
  PwrThermDmdAtStabyForCmptmt_PwrThermDmdAtStabyForCmptmt_read : Inport  Port=52
  ThermLossFromCmptmtToEnv_ThermLossFromCmptmtToEnv_read : Inport  Port=53
  TiDrvgCycPred_TiDrvgCycPred_read : Inport  Port=54
  ActvnTiForClimaCtrl_ActvnTiForClimaCtrl_read : Inport  Port=55
  AirTAftHvacDesPredAtStaby_AirTAftHvacDesPredAtStaby_read : Inport  Port=56
  ClimaAtRowSecActvNgcc_ClimaAtRowSecActvLe_read : Inport  Port=57
  ClimaAtRowSecActvNgcc_ClimaAtRowSecActvRi_read : Inport  Port=58
  CooltVlvClsd_CooltVlvClsd_read : Inport  Port=59
  HvacFlowFrntActForRowFirstAtFlr_HvacFlowFrntActForRowFirstAtFlrLe_read : Inport  Port=60
  HvacFlowFrntActForRowFirstAtFlr_HvacFlowFrntActForRowFirstAtFlrRi_read : Inport  Port=61
  HvacFlowFrntActForRowFirstAtVent_HvacFlowFrntActForRowFirstAtVentLe_read : Inport  Port=62
  HvacFlowFrntActForRowFirstAtVent_HvacFlowFrntActForRowFirstAtVentRi_read : Inport  Port=63
  HvacFlowFrntActForRowFirstAtVent_HvacFlowFrntActForRowFirstAtVentSideLe_read : Inport  Port=64
  HvacFlowFrntActForRowFirstAtVent_HvacFlowFrntActForRowFirstAtVentSideRi_read : Inport  Port=65
  HvacFlowFrntActForRowSecAtFlr_HvacFlowFrntActForRowSecAtFlrLe_read : Inport  Port=66
  HvacFlowFrntActForRowSecAtFlr_HvacFlowFrntActForRowSecAtFlrRi_read : Inport  Port=67
  HvacFlowFrntActForRowSecAtVent_HvacFlowFrntActForRowSecAtVentLe_read : Inport  Port=68
  HvacFlowFrntActForRowSecAtVent_HvacFlowFrntActForRowSecAtVentRi_read : Inport  Port=69
  function : TriggerPort  TriggerType=function-call  VariantControl=(inherit)  SampleTimeType=periodic  SampleTime=-1
  Ground_1 : Subsystem
  Ground_{5..7,9..11} (6 alike) : Ground
  Ground_2 : Subsystem
  Ground_3 : Subsystem
  Ground_4 : Subsystem
  Ground_8 : Subsystem
  Terminator_{1..69} (69 alike) : Terminator
  ClimaCoolgPwrThermPred_ClimaCoolgPwrThermPred_write : Outport
      settings: EnsureOutportIsVirtual=on
  ClimaCoolgPwrThermPredEco_ClimaCoolgPwrThermPredEco_write : Outport  Port=2
      settings: EnsureOutportIsVirtual=on
  ClimaHeatgPwrThermPred_ClimaHeatgPwrThermPred_write : Outport  Port=3
      settings: EnsureOutportIsVirtual=on
  ClimaHeatgPwrThermPredEco_ClimaHeatgPwrThermPredEco_write : Outport  Port=4
      settings: EnsureOutportIsVirtual=on
  HvacAirTEstimnAtRowAvgPredAtStaby_HvacAirTEstimnAtRowAvgPredAtStaby_write : Outport  Port=5
      settings: EnsureOutportIsVirtual=on
  PrioReqForCoolgForCmptmt_PrioReqForCoolgForCmptmt_write : Outport  Port=6
      settings: EnsureOutportIsVirtual=on
  PrioReqForHeatgForCmptmt_PrioReqForHeatgForCmptmt_write : Outport  Port=7
      settings: EnsureOutportIsVirtual=on
  PwrCnsHeatgElecPred_PwrCnsHeatgElecPred_write : Outport  Port=8
      settings: EnsureOutportIsVirtual=on
  PwrThermReqForCoolgForCmptmt_PwrThermReqForCoolgForCmptmt_write : Outport  Port=9
      settings: EnsureOutportIsVirtual=on
  PwrThermReqForHeatgForCmptmt_PwrThermReqForHeatgForCmptmt_write : Outport  Port=10
      settings: EnsureOutportIsVirtual=on
  TiToFlowNoLim_TiToFlowNoLim_write : Outport  Port=11
      settings: EnsureOutportIsVirtual=on
  wires:
  AirCdnAvl_AirCdnAvl_read/1 -> Terminator_1/1
  AirFlowLimForAirTLoActv_AirFlowLimForAirTLoActv_read/1 -> Terminator_2/1
  AirTAftHvacDes_AirTAftHvacDes_read/1 -> Terminator_3/1
  AmbTFildForClimaNgcc_AmbTFildForClima_read/1 -> Terminator_4/1
  ClimaAtRowSecActvNgcc_ClimaAtRowSecActv_read/1 -> Terminator_5/1
  ClimaCfgForHeatrTyp1_ClimaCfgForHeatrTyp1_read/1 -> Terminator_6/1
  ClimaCtrlActvNgcc_ClimaCtrlActv_read/1 -> Terminator_7/1
  ClimaTAtCmftForRowFirstLe_ClimaTAtCmftForRowFirstLe_read/1 -> Terminator_8/1
  ClimaTAtCmftForRowFirstRi_ClimaTAtCmftForRowFirstRi_read/1 -> Terminator_9/1
  ClimaTAtCmftForRowSecLe_ClimaTAtCmftForRowSecLe_read/1 -> Terminator_10/1
  ClimaTAtCmftForRowSecRi_ClimaTAtCmftForRowSecRi_read/1 -> Terminator_11/1
  CmptmtAirTEstimdAtRowFirst_CmptmtAirTEstimdAtRowFirst_read/1 -> Terminator_12/1
  CmptmtAirTEstimdAtRowSec_CmptmtAirTEstimdAtRowSec_read/1 -> Terminator_13/1
  CooltTInHeatElm_CooltTInHeatElm_read/1 -> Terminator_14/1
  EgyThermInCmptmt_EgyThermInCmptmt_read/1 -> Terminator_15/1
  EngT_Ngcc_EngT_read/1 -> Terminator_16/1
  EvaprTFrntFildForClima_EvaprTFrntFildForClima_read/1 -> Terminator_17/1
  FacForProgsToStabyForEgyCoorr_FacForProgsToStabyForEgyCoorr_read/1 -> Terminator_18/1
  HeatgPwrDmdEstimd_HeatgPwrDmdEstimd_read/1 -> Terminator_19/1
  HvacAirTEstimdAtRowFirstLe_HvacAirTEstimdAtRowFirstLe_read/1 -> Terminator_20/1
  HvacAirTEstimdAtRowFirstRi_HvacAirTEstimdAtRowFirstRi_read/1 -> Terminator_21/1
  HvacAirTEstimdAtRowSecLe_HvacAirTEstimdAtRowSecLe_read/1 -> Terminator_22/1
  HvacAirTEstimdAtRowSecRi_HvacAirTEstimdAtRowSecRi_read/1 -> Terminator_23/1
  HvacAirTReqAtRowFirstLe_HvacAirTReqAtRowFirstLe_read/1 -> Terminator_24/1
  HvacAirTReqAtRowFirstRi_HvacAirTReqAtRowFirstRi_read/1 -> Terminator_25/1
  HvacAirTReqAtRowSecLe_HvacAirTReqAtRowSecLe_read/1 -> Terminator_26/1
  HvacAirTReqAtRowSecRi_HvacAirTReqAtRowSecRi_read/1 -> Terminator_27/1
  HvacEvaprTSpFrnt_Ngcc_HvacEvaprTSpFrnt_read/1 -> Terminator_28/1
  HvacEvaprTSpFrntPredAtStaby_HvacEvaprTSpFrntPredAtStaby_read/1 -> Terminator_29/1
  HvacFlowFrntActForDefrst_HvacFlowFrntActForDefrst_read/1 -> Terminator_30/1
  HvacFlowFrntActForRowFirstAtFlr_HvacFlowFrntActForRowFirstAtFlr_read/1 -> Terminator_31/1
  HvacFlowFrntActForRowFirstAtVent_HvacFlowFrntActForRowFirstAtVent_read/1 -> Terminator_32/1
  HvacFlowFrntActForRowSecAtFlr_HvacFlowFrntActForRowSecAtFlr_read/1 -> Terminator_33/1
  HvacFlowFrntActForRowSecAtVent_HvacFlowFrntActForRowSecAtVent_read/1 -> Terminator_34/1
  HvacFlowPredForDefrstAtStaby_HvacFlowPredForDefrstAtStaby_read/1 -> Terminator_35/1
  HvacFlowPredForDefrstNoLim_HvacFlowPredForDefrstNoLim_read/1 -> Terminator_36/1
  HvacFlowPredForRowFirstAtFlrAtStaby_HvacFlowPredForRowFirstAtFlrAtStaby_read/1 -> Terminator_37/1
  HvacFlowPredForRowFirstAtFlrNoLim_HvacFlowPredForRowFirstAtFlrNoLim_read/1 -> Terminator_38/1
  HvacFlowPredForRowFirstAtVentAtStaby_HvacFlowPredForRowFirstAtVentAtStaby_read/1 -> Terminator_39/1
  HvacFlowPredForRowFirstAtVentNoLim_HvacFlowPredForRowFirstAtVentNoLim_read/1 -> Terminator_40/1
  HvacFlowPredForRowSecAtFlrAtStaby_HvacFlowPredForRowSecAtFlrAtStaby_read/1 -> Terminator_41/1
  HvacFlowPredForRowSecAtFlrNoLim_HvacFlowPredForRowSecAtFlrNoLim_read/1 -> Terminator_42/1
  HvacFlowPredForRowSecAtVentAtStaby_HvacFlowPredForRowSecAtVentAtStaby_read/1 -> Terminator_43/1
  HvacFlowPredForRowSecAtVentNoLim_HvacFlowPredForRowSecAtVentNoLim_read/1 -> Terminator_44/1
  HvacRecircRatForEgyCalcn_HvacRecircRatForEgyCalcn_read/1 -> Terminator_45/1
  HvacRecircRatPredAtStaby_HvacRecircRatPredAtStaby_read/1 -> Terminator_46/1
  HvacRecircRatReq_HvacRecircRatReq_read/1 -> Terminator_47/1
  HvacTotFlowForCmptmt_HvacTotFlowForCmptmt_read/1 -> Terminator_48/1
  HvBattCoolgPwrHeatgMgrThermPred_HvBattCoolgPwrHeatgMgrThermPred_read/1 -> Terminator_49/1
  PwrThermAllwdForCoolgForCmptmt_PwrThermAllwdForCoolgForCmptmt_read/1 -> Terminator_50/1
  PwrThermAllwdForHeatForCmptmt_PwrThermAllwdForHeatForCmptmt_read/1 -> Terminator_51/1
  PwrThermDmdAtStabyForCmptmt_PwrThermDmdAtStabyForCmptmt_read/1 -> Terminator_52/1
  ThermLossFromCmptmtToEnv_ThermLossFromCmptmtToEnv_read/1 -> Terminator_53/1
  TiDrvgCycPred_TiDrvgCycPred_read/1 -> Terminator_54/1
  ActvnTiForClimaCtrl_ActvnTiForClimaCtrl_read/1 -> Terminator_55/1
  AirTAftHvacDesPredAtStaby_AirTAftHvacDesPredAtStaby_read/1 -> Terminator_56/1
  ClimaAtRowSecActvNgcc_ClimaAtRowSecActvLe_read/1 -> Terminator_57/1
  ClimaAtRowSecActvNgcc_ClimaAtRowSecActvRi_read/1 -> Terminator_58/1
  CooltVlvClsd_CooltVlvClsd_read/1 -> Terminator_59/1
  HvacFlowFrntActForRowFirstAtFlr_HvacFlowFrntActForRowFirstAtFlrLe_read/1 -> Terminator_60/1
  HvacFlowFrntActForRowFirstAtFlr_HvacFlowFrntActForRowFirstAtFlrRi_read/1 -> Terminator_61/1
  HvacFlowFrntActForRowFirstAtVent_HvacFlowFrntActForRowFirstAtVentLe_read/1 -> Terminator_62/1
  HvacFlowFrntActForRowFirstAtVent_HvacFlowFrntActForRowFirstAtVentRi_read/1 -> Terminator_63/1
  HvacFlowFrntActForRowFirstAtVent_HvacFlowFrntActForRowFirstAtVentSideLe_read/1 -> Terminator_64/1
  HvacFlowFrntActForRowFirstAtVent_HvacFlowFrntActForRowFirstAtVentSideRi_read/1 -> Terminator_65/1
  HvacFlowFrntActForRowSecAtFlr_HvacFlowFrntActForRowSecAtFlrLe_read/1 -> Terminator_66/1
  HvacFlowFrntActForRowSecAtFlr_HvacFlowFrntActForRowSecAtFlrRi_read/1 -> Terminator_67/1
  HvacFlowFrntActForRowSecAtVent_HvacFlowFrntActForRowSecAtVentLe_read/1 -> Terminator_68/1
  HvacFlowFrntActForRowSecAtVent_HvacFlowFrntActForRowSecAtVentRi_read/1 -> Terminator_69/1
  Ground_1/1(Out1) -> ClimaCoolgPwrThermPred_ClimaCoolgPwrThermPred_write/1
  Ground_2/1(Out1) -> ClimaCoolgPwrThermPredEco_ClimaCoolgPwrThermPredEco_write/1
  Ground_3/1(Out1) -> ClimaHeatgPwrThermPred_ClimaHeatgPwrThermPred_write/1
  Ground_4/1(Out1) -> ClimaHeatgPwrThermPredEco_ClimaHeatgPwrThermPredEco_write/1
  Ground_5/1 -> HvacAirTEstimnAtRowAvgPredAtStaby_HvacAirTEstimnAtRowAvgPredAtStaby_write/1
  Ground_6/1 -> PrioReqForCoolgForCmptmt_PrioReqForCoolgForCmptmt_write/1
  Ground_7/1 -> PrioReqForHeatgForCmptmt_PrioReqForHeatgForCmptmt_write/1
  Ground_8/1(Out1) -> PwrCnsHeatgElecPred_PwrCnsHeatgElecPred_write/1
  Ground_9/1 -> PwrThermReqForCoolgForCmptmt_PwrThermReqForCoolgForCmptmt_write/1
  Ground_10/1 -> PwrThermReqForHeatgForCmptmt_PwrThermReqForHeatgForCmptmt_write/1
  Ground_11/1 -> TiToFlowNoLim_TiToFlowNoLim_write/1

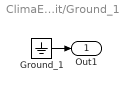
== system ClimaEgyCoorr/ClimaEgyCoorr_Init/Ground_1 ==
  Ground_1 : Ground
  Out1 : Outport  OutDataTypeStr=Bus: ClimaCoolgPwrThermPred  PortDimensions=1
  wires:
  Ground_1/1 -> Out1/1

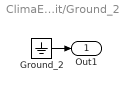
== system ClimaEgyCoorr/ClimaEgyCoorr_Init/Ground_2 ==
  Ground_2 : Ground
  Out1 : Outport  OutDataTypeStr=Bus: ClimaCoolgPwrThermPredEcoRec  PortDimensions=1
  wires:
  Ground_2/1 -> Out1/1

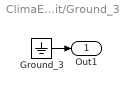
== system ClimaEgyCoorr/ClimaEgyCoorr_Init/Ground_3 ==
  Ground_3 : Ground
  Out1 : Outport  OutDataTypeStr=Bus: ClimaHeatgPwrThermPred  PortDimensions=1
  wires:
  Ground_3/1 -> Out1/1

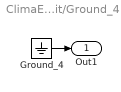
== system ClimaEgyCoorr/ClimaEgyCoorr_Init/Ground_4 ==
  Ground_4 : Ground
  Out1 : Outport  OutDataTypeStr=Bus: ClimaHeatgPwrThermPredEcoRec  PortDimensions=1
  wires:
  Ground_4/1 -> Out1/1

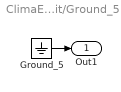
== system ClimaEgyCoorr/ClimaEgyCoorr_Init/Ground_5 ==
  Ground_5 : Ground
  Out1 : Outport  OutDataTypeStr=Bus: PwrCnsHeatgElecPred  PortDimensions=1
  wires:
  Ground_5/1 -> Out1/1

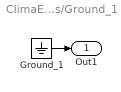
== system ClimaEgyCoorr/ClimaEgyCoorr_Runnable_sys/Ground_1 ==
  Ground_1 : Ground
  Out1 : Outport  OutDataTypeStr=Bus: ClimaCoolgPwrThermPred  PortDimensions=1
  wires:
  Ground_1/1 -> Out1/1

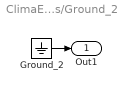
== system ClimaEgyCoorr/ClimaEgyCoorr_Runnable_sys/Ground_2 ==
  Ground_2 : Ground
  Out1 : Outport  OutDataTypeStr=Bus: ClimaCoolgPwrThermPredEcoRec  PortDimensions=1
  wires:
  Ground_2/1 -> Out1/1

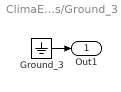
== system ClimaEgyCoorr/ClimaEgyCoorr_Runnable_sys/Ground_3 ==
  Ground_3 : Ground
  Out1 : Outport  OutDataTypeStr=Bus: ClimaHeatgPwrThermPred  PortDimensions=1
  wires:
  Ground_3/1 -> Out1/1

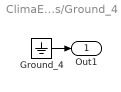
== system ClimaEgyCoorr/ClimaEgyCoorr_Runnable_sys/Ground_4 ==
  Ground_4 : Ground
  Out1 : Outport  OutDataTypeStr=Bus: ClimaHeatgPwrThermPredEcoRec  PortDimensions=1
  wires:
  Ground_4/1 -> Out1/1

== system ClimaEgyCoorr/ClimaEgyCoorr_Runnable_sys/Ground_8 ==
  Ground_8 : Ground
  Out1 : Outport  OutDataTypeStr=Bus: PwrCnsHeatgElecPred  PortDimensions=1
  wires:
  Ground_8/1 -> Out1/1
